simulink model "TosAeb"  (R2021a)
settings: Solver=FixedStepDiscrete  StartTime=0.0  StopTime=inf  FixedStep=0.01  RelTol=1e-3
undefined symbols (not defined in the file or in its folder): ACCModSts, AccSts1, AEB_ObjectMovingOrientation, AEB_ObjectMovingState, CN_BTCActive, CN_CurrentGearR, CN_CurrentGearR_DHT, CN_PTCActive, CN_VDCActive, CN_VSC_EspOff, CN_VSC_FCW_AEB_Error, CN_VSC_FCW_AEB_NoDispay, CN_VSC_FCW_AEB_PerfmDgdtn, CN_VSC_FCW_AEB_TempUnAvlbl, CN_VSC_VehStandstill, CNaccelAvoidMax, CNaccelAvoidMin, CNbrakePedalPress, CNcomfortStrLeftAccelMax, CNcomfortStrLeftAccelMin, CNcomfortStrRightAccelMax, CNcomfortStrRightAccelMin, CNDrvDoorOpen, CNDrvModeEco, CNDrvModeNorm, CNDrvModeOther, CNDrvModeSport, CNDrvSBRreminder, CNNull, CNobjInvalidID, CNPassDoorOpen, CNPassSBRreminder, CNPEBSAccelNegInf, CNPEBSAccelNull, CNPEBSTimeInf, CNPEBSTimeNull, CNSTOPMAX, CNSTOPNULL, CNsubjWidth, CNSwtOFF, CNSwtON, CNTTCMAX, CNTTCNULL, CNVehConfigAEBCycNone, CNVehConfigAEBNone, CNVehConfigAEBPedNone, CNVehConfigFCWNone, CNVehConfigJANone, CollisionSeverity, FALSE, FCWSnvty, JACycObjCalcInfoDefaultParams, JAPedObjCalcInfoDefaultParams, JAVehObjCalcInfoDefaultParams, latCycObjCalcInfoDefaultParams, latPedObjCalcInfoDefaultParams, longObjCalcInfoDefaultParams, ModSts, movingStatus, ObjectType ... (+1112)
libraries: simulink x3031, AEBSFunctionLib x50, Ta_Library x33, AEBSStateMachineLib x23, Mylibrary x12, simulink_extras x7, ObjSelect_lib x3, dspstat3 x2
(part 54 of 102)

== system TosAeb/FcwAebJa_Step_sys/ALG_AEBSystem/ALG_AEBController/AEB_FuncCondCalcApp/PedFunctionCondCalc/pedABASuppAborCond/pedABASuppCondCalc/pedABASituationRelActiveCondCalc/pedABASituationRelSuppCondCalc ==
  latCollisionSeverity : Inport
  subjCurvTraj : Inport  Port=2
  subjLatAccel : Inport  Port=3
  colliisonRiskProb : Inport  Port=4
  ObjectTypeCameraConfirmed : Inport  Port=5
  pedLatVyCond4ABA : Inport  Port=6
  objLongDistCond4ABA : Inport  Port=7
  Compare To Constant1 : simulink/Logic and Bit Operations/Compare To Constant  relop===  const=CollisionSeverity.None  OutDataTypeStr=boolean
  Compare To Constant10 : simulink/Logic and Bit Operations/Compare To Constant  relop=>=  const=P_AEB_Func_pedABAColliisonRiskProbSupThres4Radar  OutDataTypeStr=boolean
  Compare To Constant11 : simulink/Logic and Bit Operations/Compare To Constant  relop===  const=FALSE  OutDataTypeStr=boolean
  Compare To Constant2 : simulink/Logic and Bit Operations/Compare To Constant  relop=>  const=P_AEB_Func_pedABASubjCurvTrajSupThres  OutDataTypeStr=boolean
  Compare To Constant3 : simulink/Logic and Bit Operations/Compare To Constant  relop=>  const=P_AEB_Func_pedABASubjLatAccelSupThres  OutDataTypeStr=boolean
  Compare To Constant4 : simulink/Logic and Bit Operations/Compare To Constant  relop===  const=FALSE  OutDataTypeStr=boolean
  Compare To Constant7 : simulink/Logic and Bit Operations/Compare To Constant  relop===  const=FALSE  OutDataTypeStr=boolean
  Compare To Constant8 : simulink/Logic and Bit Operations/Compare To Constant  relop===  const=TRUE  OutDataTypeStr=boolean
  Compare To Constant9 : simulink/Logic and Bit Operations/Compare To Constant  relop=>=  const=P_AEB_Func_pedABAColliisonRiskProbSupThres4Fusion  OutDataTypeStr=boolean
  Logical Operator1 : Logic  Operator=OR  AllPortsSameDT=off  OutDataTypeStr=boolean
  Logical Operator2 : Logic  Operator=OR  Inputs=5  AllPortsSameDT=off  OutDataTypeStr=boolean
  Switch : Switch  Criteria=u2 ~= 0
  pedABASituationRelSuppCondFulfilled : Outport
  wires:
  Compare To Constant8/1 -> Switch/2
  colliisonRiskProb/1 -> Compare To Constant9/1, Compare To Constant10/1
  Switch/1 -> Compare To Constant11/1
  Compare To Constant10/1 -> Switch/3
  Compare To Constant9/1 -> Switch/1
  ObjectTypeCameraConfirmed/1 -> Compare To Constant8/1
  latCollisionSeverity/1 -> Compare To Constant1/1
  objLongDistCond4ABA/1 -> Compare To Constant7/1
  Compare To Constant4/1 -> Logical Operator2/4  "AEB_PedLatVySuppCond4ABA"
  subjLatAccel/1 -> Compare To Constant3/1
  Compare To Constant7/1 -> Logical Operator2/5  "AEB_PedLongDistSuppCond4ABA"
  Compare To Constant11/1 -> Logical Operator2/3  "pedCritTriggerReleventSuppCondFulfilled"
  Logical Operator1/1 -> Logical Operator2/2  "brakeReleventSuppCondFulfilled"
  Compare To Constant1/1 -> Logical Operator2/1  "pedCollisionSeverityRelSuppCondFulfilled"
  subjCurvTraj/1 -> Compare To Constant2/1
  Logical Operator2/1 -> pedABASituationRelSuppCondFulfilled/1
  Compare To Constant2/1 -> Logical Operator1/1
  pedLatVyCond4ABA/1 -> Compare To Constant4/1
  Compare To Constant3/1 -> Logical Operator1/2

== system TosAeb/FcwAebJa_Step_sys/ALG_AEBSystem/ALG_AEBController/AEB_FuncCondCalcApp/PedFunctionCondCalc/pedABASuppAborCond/pedABASuppCondCalc/pedABASituationRelActiveCondCalc/vyCorrCalc ==
  ObjectTypeCameraConfirmed : Inport
  vy : Inport  Port=2
  Abs : Abs
  Compare To Constant10 : simulink/Logic and Bit Operations/Compare To Constant  relop=>=  const=P_AEB_Func_pedABAVyThres4Radar  OutDataTypeStr=boolean
  Compare To Constant8 : simulink/Logic and Bit Operations/Compare To Constant  relop===  const=TRUE  OutDataTypeStr=boolean
  Compare To Constant9 : simulink/Logic and Bit Operations/Compare To Constant  relop=>=  const=P_AEB_Func_pedABAVyThres4Fusion  OutDataTypeStr=boolean
  Switch : Switch  Criteria=u2 ~= 0
  objLatVyCond4ABA : Outport
  wires:
  Abs/1 -> Compare To Constant10/1, Compare To Constant9/1
  Compare To Constant10/1 -> Switch/3
  ObjectTypeCameraConfirmed/1 -> Compare To Constant8/1
  Compare To Constant8/1 -> Switch/2
  Switch/1 -> objLatVyCond4ABA/1
  Compare To Constant9/1 -> Switch/1
  vy/1 -> Abs/1  "vy"

== system TosAeb/FcwAebJa_Step_sys/ALG_AEBSystem/ALG_AEBController/AEB_FuncCondCalcApp/PedFunctionCondCalc/pedAEBfullSuppAborCond/pedAEBfullSuppCondCalc/pedAEBfullSituationRelSuppCondCalc/dy_ConfirmCalc ==
  note: P_AEB_Func_pedAEBfullDy
  note: [11.1;16.67]
      | [0.12;0.1]
  subjVel : Inport
  dxFrontBumper : Inport  Port=2
  dy : Inport  Port=3
  1-D Lookup Table : Lookup_n-D  NumberOfTableDimensions=1  BreakpointsForDimension1=P_AEB_Func_pedAEBfullDyConfirmX  Table=P_AEB_Func_pedAEBfullDyConfirmY  ExtrapMethod=Clip  InternalRulePriority=Speed  RndMeth=Simplest  InputSameDT=on
  Add : Sum  Inputs=+++
  Constant : Constant  Value=P_AEB_Func_pedAEBfullDyOffset
  Constant1 : Constant  Value=CNsubjWidth
  Gain : Gain  Gain=0.5
  Product : Product
  Relational Operator : RelationalOperator  Operator=<=  OutDataTypeStr=boolean  RndMeth=Simplest
  dy_Confirm : Outport
  wires:
  Relational Operator/1 -> dy_Confirm/1
  Add/1 -> Relational Operator/2
  dy/1 -> Relational Operator/1
  Product/1 -> Add/3
  Constant/1 -> Add/2
  Gain/1 -> Add/1
  Constant1/1 -> Gain/1
  dxFrontBumper/1 -> Product/2
  1-D Lookup Table/1 -> Product/1
  subjVel/1 -> 1-D Lookup Table/1

== system TosAeb/FcwAebJa_Step_sys/ALG_AEBSystem/ALG_AEBController/AEB_FuncCondCalcApp/PedFunctionCondCalc/pedAEBfullSuppAborCond/pedAEBfullSuppCondCalc/pedAEBfullSituationRelSuppCondCalc/latDistCondCalc ==
  dy : Inport
  FcwHMISetting : Inport  Port=2
  subjVel : Inport  Port=3
  vy : Inport  Port=4
  Abs : Abs
  LateralDistanceThresHMISettingEarly : Subsystem  TreatAsAtomicUnit=on
  LateralDistanceThresHMISettingLate : Subsystem  TreatAsAtomicUnit=on
  Merge : Merge
  Relational Operator : RelationalOperator  Operator=<=  OutDataTypeStr=boolean  RndMeth=Simplest
  Switch Case : SwitchCase  CaseConditions={[FCWSnvty.HMISettingEarly,FCWSnvty.HMISettingMiddle]}
  latDistCond : Outport
  wires:
  LateralDistanceThresHMISettingEarly/1(latDistThres) -> Merge/1
  FcwHMISetting/1 -> Switch Case/1
  Abs/1 -> Relational Operator/1
  Switch Case/1 -> LateralDistanceThresHMISettingEarly/Action
  Merge/1 -> Relational Operator/2  "latDistThres"
  dy/1 -> Abs/1
  Relational Operator/1 -> latDistCond/1
  Switch Case/2 -> [HMISettingLate]
  LateralDistanceThresHMISettingLate/1(latDistThres) -> Merge/2
  subjVel/1 -> LateralDistanceThresHMISettingEarly/1(subjVel)
  vy/1 -> LateralDistanceThresHMISettingLate/1(vy)
  [HMISettingLate] -> LateralDistanceThresHMISettingLate/Action

== system TosAeb/FcwAebJa_Step_sys/ALG_AEBSystem/ALG_AEBController/AEB_FuncCondCalcApp/PedFunctionCondCalc/pedAEBfullSuppAborCond/pedAEBfullSuppCondCalc/pedAEBfullSituationRelSuppCondCalc/longDistCond4fullBrkCalc ==
  dxFrontBumper : Inport
  distSecure : Inport  Port=2
  distBraking : Inport  Port=3
  subjVel : Inport  Port=4
  Add : Sum
  Constant : Constant  Value=P_AEB_Func_pedAEBfullLongDistTimeOffset
  Constant1 : Constant  Value=0.1  OutDataTypeStr=single
  Product : Product
  Relational Operator : RelationalOperator  Operator=<=  OutDataTypeStr=boolean  RndMeth=Simplest
  Relational Operator1 : RelationalOperator  Operator=<=  OutDataTypeStr=boolean  RndMeth=Simplest
  Terminator : Terminator
  longDistCond4fullBrk : Outport
  longDistMinCond4Brake : Outport  Port=2
  wires:
  Constant/1 -> Product/2
  subjVel/1 -> Product/1
  dxFrontBumper/1 -> Relational Operator/1, Relational Operator1/1
  Relational Operator1/1 -> longDistMinCond4Brake/1
  Add/1 -> Relational Operator/2
  distBraking/1 -> Add/2
  distSecure/1 -> Add/1
  Relational Operator/1 -> longDistCond4fullBrk/1
  Constant1/1 -> Relational Operator1/2
  Product/1 -> Terminator/1

== system TosAeb/FcwAebJa_Step_sys/ALG_AEBSystem/ALG_AEBController/AEB_FuncCondCalcApp/PedFunctionCondCalc/pedAEBfullSuppAborCond/pedAEBfullSuppCondCalc/pedAEBfullSituationRelSuppCondCalc/objLatVyCond ==
  ObjectTypeCameraConfirmed : Inport
  vy : Inport  Port=2
  dy : Inport  Port=3
  Constant : Constant  Value=P_AEB_Func_pedAEBfullVyMaxThres
  Constant1 : Constant  Value=P_AEB_Func_pedAEBfullDyInnerAreaThres
  Constant2 : Constant  Value=P_AEB_Func_pedAEBfullDyMiddleAreaThres
  Constant3 : Constant  Value=P_AEB_Func_pedAEBfullVyInnerAreaThres4Fusion
  Constant4 : Constant  Value=P_AEB_Func_pedAEBfullVyMiddleAreaThres4Fusion
  Constant5 : Constant  Value=P_AEB_Func_pedAEBfullVyOuterAreaThres4Fusion
  Constant6 : Constant  Value=P_AEB_Func_pedAEBfullVyInnerAreaThres4Radar
  Constant7 : Constant  Value=P_AEB_Func_pedAEBfullVyMiddleAreaThres4Radar
  Constant8 : Constant  Value=P_AEB_Func_pedAEBfullVyOuterAreaThres4Radar
  objLatVyCond4FullBrakeCalc : Stateflow chart  TreatAsAtomicUnit=on
  objLatVyCond4FullBrake : Outport
  wires:
  Constant8/1 -> objLatVyCond4FullBrakeCalc/12(P_pedAEBfullVyOuterAreaThres4Radar)
  Constant7/1 -> objLatVyCond4FullBrakeCalc/11(P_pedAEBfullVyMiddleAreaThres4Radar)
  Constant6/1 -> objLatVyCond4FullBrakeCalc/10(P_pedAEBfullVyInnerAreaThres4Radar)
  Constant5/1 -> objLatVyCond4FullBrakeCalc/9(P_pedAEBfullVyOuterAreaThres4Fusion)
  Constant4/1 -> objLatVyCond4FullBrakeCalc/8(P_pedAEBfullVyMiddleAreaThres4Fusion)
  Constant3/1 -> objLatVyCond4FullBrakeCalc/7(P_pedAEBfullVyInnerAreaThres4Fusion)
  Constant2/1 -> objLatVyCond4FullBrakeCalc/6(P_pedAEBfullDyMiddleAreaThres)
  Constant1/1 -> objLatVyCond4FullBrakeCalc/5(P_pedAEBfullDyInnerAreaThres)
  Constant/1 -> objLatVyCond4FullBrakeCalc/4(P_pedAEBfullVyMaxThres)
  vy/1 -> objLatVyCond4FullBrakeCalc/3(vy)
  ObjectTypeCameraConfirmed/1 -> objLatVyCond4FullBrakeCalc/1(objFusionType)
  dy/1 -> objLatVyCond4FullBrakeCalc/2(dy)
  objLatVyCond4FullBrakeCalc/1(objLatVyCond4FullBrake) -> objLatVyCond4FullBrake/1

== system TosAeb/FcwAebJa_Step_sys/ALG_AEBSystem/ALG_AEBController/AEB_FuncCondCalcApp/PedFunctionCondCalc/pedAEBfullSuppAborCond/pedAEBfullSuppCondCalc/pedAEBfullSituationRelSuppCondCalc/pedAEBfullSituationRelSuppCond ==
  latCollisionSeverity : Inport
  subjCurvTraj : Inport  Port=2
  subjLatAccel : Inport  Port=3
  pedCollisionProbFlg : Inport  Port=4
  pedCollisionRisk : Inport  Port=5
  ObjectTypeCameraConfirmed : Inport  Port=6
  objLatVyCond4FullBrake : Inport  Port=7
  latDistCond : Inport  Port=8
  objLongDistCond4FullBrake : Inport  Port=9
  distMinCond4FullBrake : Inport  Port=10
  Compare To Constant : simulink/Logic and Bit Operations/Compare To Constant  relop===  const=TRUE  OutDataTypeStr=boolean
  Compare To Constant1 : simulink/Logic and Bit Operations/Compare To Constant  relop===  const=CollisionSeverity.None  OutDataTypeStr=boolean
  Compare To Constant{4,6..7,9..10} (5 alike) : simulink/Logic and Bit Operations/Compare To Constant  relop===  const=FALSE  OutDataTypeStr=boolean
  Compare To Constant11 : simulink/Logic and Bit Operations/Compare To Constant  relop===  const=TRUE  OutDataTypeStr=boolean
  Compare To Constant12 : simulink/Logic and Bit Operations/Compare To Constant  relop=>  const=P_AEB_Func_pedAEBfullColliisonRiskProbSupThres4Fusion  OutDataTypeStr=boolean
  Compare To Constant13 : simulink/Logic and Bit Operations/Compare To Constant  relop=>  const=P_AEB_Func_pedAEBfullColliisonRiskProbSupThres4Radar  OutDataTypeStr=boolean
  Compare To Constant2 : simulink/Logic and Bit Operations/Compare To Constant  relop=>  const=P_AEB_Func_pedAEBfullSubjCurvTrajSupThres  OutDataTypeStr=boolean
  Compare To Constant3 : simulink/Logic and Bit Operations/Compare To Constant  relop=>  const=P_AEB_Func_pedAEBfullSubjLatAccelSupThres  OutDataTypeStr=boolean
  Compare To Constant5 : simulink/Logic and Bit Operations/Compare To Constant  relop===  const=CollisionSeverity.Low  OutDataTypeStr=boolean
  Compare To Constant8 : simulink/Logic and Bit Operations/Compare To Constant  relop===  const=CollisionSeverity.Medium  OutDataTypeStr=boolean
  Logical Operator1 : Logic  Operator=OR  AllPortsSameDT=off  OutDataTypeStr=boolean
  Logical Operator2 : Logic  Operator=OR  Inputs=7  AllPortsSameDT=off  OutDataTypeStr=boolean
  Logical Operator3 : Logic  Operator=OR  Inputs=3  AllPortsSameDT=off  OutDataTypeStr=boolean
  Logical Operator4 : Logic  Operator=OR  AllPortsSameDT=off  OutDataTypeStr=boolean
  Switch : Switch  Criteria=u2 ~= 0
  pedAEBfullSituationRelSuppCondFulfilled : Outport
  wires:
  Compare To Constant13/1 -> Switch/3
  Compare To Constant12/1 -> Switch/1
  Switch/1 -> Compare To Constant6/1
  pedCollisionRisk/1 -> Compare To Constant12/1, Compare To Constant13/1
  Compare To Constant/1 -> Switch/2
  ObjectTypeCameraConfirmed/1 -> Compare To Constant/1
  Compare To Constant11/1 -> Logical Operator2/7  "AEB_PedLongDistMinSuppCond4FullBrake"
  Compare To Constant7/1 -> Logical Operator2/6  "AEB_PedLongDistSuppCond4FullBrake"
  Compare To Constant9/1 -> Logical Operator2/5  "AEB_PedLatDistSuppCond4FullBrake"
  distMinCond4FullBrake/1 -> Compare To Constant11/1
  latCollisionSeverity/1 -> Compare To Constant8/1, Compare To Constant5/1, Compare To Constant1/1
  objLongDistCond4FullBrake/1 -> Compare To Constant7/1
  Compare To Constant4/1 -> Logical Operator2/4  "AEB_PedLatVySuppCond4FullBrake"
  subjLatAccel/1 -> Compare To Constant3/1
  Logical Operator4/1 -> Logical Operator2/3  "pedCritTriggerReleventSuppCondFulfilled"
  Logical Operator1/1 -> Logical Operator2/2  "SubjMovingReleventSuppCondFulfilled"
  Logical Operator3/1 -> Logical Operator2/1  "pedCollisionDgrLvlRelSuppCondFulfilled"
  subjCurvTraj/1 -> Compare To Constant2/1
  Logical Operator2/1 -> pedAEBfullSituationRelSuppCondFulfilled/1
  Compare To Constant2/1 -> Logical Operator1/1
  objLatVyCond4FullBrake/1 -> Compare To Constant4/1
  Compare To Constant3/1 -> Logical Operator1/2
  Compare To Constant1/1 -> Logical Operator3/1
  Compare To Constant5/1 -> Logical Operator3/2
  Compare To Constant8/1 -> Logical Operator3/3
  latDistCond/1 -> Compare To Constant9/1
  pedCollisionProbFlg/1 -> Compare To Constant10/1
  Compare To Constant6/1 -> Logical Operator4/2
  Compare To Constant10/1 -> Logical Operator4/1

== system TosAeb/FcwAebJa_Step_sys/ALG_AEBSystem/ALG_AEBController/AEB_FuncCondCalcApp/PedFunctionCondCalc/pedAEBfullSuppAborCond/pedAEBfullSuppCondCalc/pedAEBfullSituationRelSuppCondCalc/vy_ConfirmCalc ==
  vy : Inport
  dy : Inport  Port=2
  1-D Lookup Table : Lookup_n-D  NumberOfTableDimensions=1  BreakpointsForDimension1=[0.45;2]  Table=[0.6;1.15]  ExtrapMethod=Clip  InternalRulePriority=Speed  RndMeth=Simplest  InputSameDT=on
  Relational Operator : RelationalOperator  Operator=>  OutDataTypeStr=boolean  RndMeth=Simplest
  vy_Confirm : Outport
  wires:
  dy/1 -> 1-D Lookup Table/1
  vy/1 -> Relational Operator/1
  Relational Operator/1 -> vy_Confirm/1
  1-D Lookup Table/1 -> Relational Operator/2

== system TosAeb/FcwAebJa_Step_sys/ALG_AEBSystem/ALG_AEBController/AEB_FuncCondCalcApp/PedFunctionCondCalc/pedAEBfullSuppAborCond/pedAEBfullSuppCondCalc/pedAEBfullVehRelSuppCondCalc/brakeReleventSuppCond ==
  VehConfigIbooster : Inport
  ESP_MasterCylindBrakePressValid : Inport  Port=2
  ESP_MasterCylindBrakePress : Inport  Port=3
  Compare To Constant : simulink/Logic and Bit Operations/Compare To Constant  relop===  const=FALSE  OutDataTypeStr=boolean
  If : If  IfExpression=u1
  Merge : Merge
  brakeReleventSuppCond_ESP : Subsystem  VariantControl=(default)
  brakeReleventSuppCond_IBooster : Subsystem  VariantControl=P_AEB_PowerTrainConfig == 1
  brakeReleventSuppCond : Outport
  wires:
  ESP_MasterCylindBrakePress/1 -> brakeReleventSuppCond_ESP/2(ESP_MasterCylindBrakePress)
  ESP_MasterCylindBrakePressValid/1 -> brakeReleventSuppCond_ESP/1(ESP_MasterCylindBrakePressValid)
  Merge/1 -> brakeReleventSuppCond/1
  brakeReleventSuppCond_IBooster/1(brakeReleventSuppCond) -> Merge/2
  brakeReleventSuppCond_ESP/1(brakeReleventSuppCond) -> Merge/1
  If/2 -> brakeReleventSuppCond_IBooster/Action
  If/1 -> brakeReleventSuppCond_ESP/Action
  Compare To Constant/1 -> If/1
  VehConfigIbooster/1 -> Compare To Constant/1

== system TosAeb/FcwAebJa_Step_sys/ALG_AEBSystem/ALG_AEBController/AEB_FuncCondCalcApp/PedFunctionCondCalc/pedPreWarningSuppAborCond/pedPreWarningSuppCondCalc/pedPreWarningSituationRelActiveCondCalc/PreWarningMaxTimeCalc ==
  FcwHMISetting : Inport
  subjVel : Inport  Port=2
  dxFrontBumper : Inport  Port=3
  Compare To Constant : simulink/Logic and Bit Operations/Compare To Constant  relop===  const=FCWSnvty.HMISettingEarly  OutDataTypeStr=boolean
  Compare To Constant1 : simulink/Logic and Bit Operations/Compare To Constant  relop===  const=FCWSnvty.HMISettingMiddle  OutDataTypeStr=boolean
  Constant : Constant  Value=P_AEB_Func_pedPrewarningLongDistTimeOffsetHmiEarlyMid
  Constant1 : Constant  Value=P_AEB_Func_pedPrewarningLongDistTimeOffsetHmiLate
  Logical Operator : Logic  Operator=OR  AllPortsSameDT=off  OutDataTypeStr=boolean
  Product : Product
  Relational Operator : RelationalOperator  Operator=<=  OutDataTypeStr=boolean  RndMeth=Simplest
  Switch : Switch  Criteria=u2 ~= 0
  longDistCond4PreWarning : Outport
  wires:
  FcwHMISetting/1 -> Compare To Constant1/1, Compare To Constant/1
  Compare To Constant/1 -> Logical Operator/1
  Compare To Constant1/1 -> Logical Operator/2
  Logical Operator/1 -> Switch/2
  Constant/1 -> Switch/1
  Constant1/1 -> Switch/3
  Switch/1 -> Product/1
  subjVel/1 -> Product/2
  Product/1 -> Relational Operator/2
  dxFrontBumper/1 -> Relational Operator/1
  Relational Operator/1 -> longDistCond4PreWarning/1

== system TosAeb/FcwAebJa_Step_sys/ALG_AEBSystem/ALG_AEBController/AEB_FuncCondCalcApp/PedFunctionCondCalc/pedPreWarningSuppAborCond/pedPreWarningSuppCondCalc/pedPreWarningSituationRelActiveCondCalc/longDistMinCond4PreWarningCalc ==
  dxFrontBumper : Inport
  subjVel : Inport  Port=2
  Constant : Constant
  Product : Product
  Relational Operator : RelationalOperator  Operator=<=  OutDataTypeStr=boolean  RndMeth=Simplest
  longDistMinCond4PreWarning : Outport
  wires:
  Relational Operator/1 -> longDistMinCond4PreWarning/1
  dxFrontBumper/1 -> Relational Operator/1
  subjVel/1 -> Product/1
  Constant/1 -> Product/2
  Product/1 -> Relational Operator/2

== system TosAeb/FcwAebJa_Step_sys/ALG_AEBSystem/ALG_AEBController/AEB_FuncCondCalcApp/PedFunctionCondCalc/pedPreWarningSuppAborCond/pedPreWarningSuppCondCalc/pedPreWarningSituationRelActiveCondCalc/pedPreWarningSituationRelSuppCond ==
  latCollisionSeverity : Inport
  subjCurvTraj : Inport  Port=2
  subjLatAccel : Inport  Port=3
  colliisonRiskProb : Inport  Port=4
  ObjectTypeCameraConfirmed : Inport  Port=5
  pedLatVyCond4Prewarning : Inport  Port=6
  longDistCond4PreWarning : Inport  Port=7
  longDistMinCond4PreWarning : Inport  Port=8
  Compare To Constant1 : simulink/Logic and Bit Operations/Compare To Constant  relop===  const=CollisionSeverity.None  OutDataTypeStr=boolean
  Compare To Constant10 : simulink/Logic and Bit Operations/Compare To Constant  relop=>=  const=P_AEB_Func_pedPrewarningColliisonRiskProbSupThres4Radar  OutDataTypeStr=boolean
  Compare To Constant{4..5,7,11} (4 alike) : simulink/Logic and Bit Operations/Compare To Constant  relop===  const=FALSE  OutDataTypeStr=boolean
  Compare To Constant2 : simulink/Logic and Bit Operations/Compare To Constant  relop=>  const=P_AEB_Func_pedPrewarningSubjCurvTrajSupThres  OutDataTypeStr=boolean
  Compare To Constant3 : simulink/Logic and Bit Operations/Compare To Constant  relop=>  const=P_AEB_Func_pedPrewarningSubjLatAccelSupThres  OutDataTypeStr=boolean
  Compare To Constant8 : simulink/Logic and Bit Operations/Compare To Constant  relop===  const=TRUE  OutDataTypeStr=boolean
  Compare To Constant9 : simulink/Logic and Bit Operations/Compare To Constant  relop=>=  const=P_AEB_Func_pedPrewarningColliisonRiskProbSupThres4Fusion  OutDataTypeStr=boolean
  Logical Operator1 : Logic  Operator=OR  AllPortsSameDT=off  OutDataTypeStr=boolean
  Logical Operator2 : Logic  Operator=OR  Inputs=6  AllPortsSameDT=off  OutDataTypeStr=boolean
  Switch : Switch  Criteria=u2 ~= 0
  pedPreWarningSituationRelSuppCondFulfilled : Outport
  wires:
  Compare To Constant5/1 -> Logical Operator2/6  "AEB_PedLongDistMinSuppCond4PreWarning"
  Compare To Constant7/1 -> Logical Operator2/5  "AEB_PedLongDistSuppCond4PreWarning"
  Compare To Constant4/1 -> Logical Operator2/4  "AEB_PedLatVySuppCond4Prewarning"
  Compare To Constant11/1 -> Logical Operator2/3
  Compare To Constant10/1 -> Switch/3
  Switch/1 -> Compare To Constant11/1
  ObjectTypeCameraConfirmed/1 -> Compare To Constant8/1
  longDistCond4PreWarning/1 -> Compare To Constant7/1
  longDistMinCond4PreWarning/1 -> Compare To Constant5/1
  Compare To Constant9/1 -> Switch/1
  Compare To Constant8/1 -> Switch/2
  colliisonRiskProb/1 -> Compare To Constant10/1, Compare To Constant9/1
  Compare To Constant3/1 -> Logical Operator1/2
  pedLatVyCond4Prewarning/1 -> Compare To Constant4/1
  Compare To Constant2/1 -> Logical Operator1/1
  Logical Operator2/1 -> pedPreWarningSituationRelSuppCondFulfilled/1
  subjCurvTraj/1 -> Compare To Constant2/1
  Compare To Constant1/1 -> Logical Operator2/1
  Logical Operator1/1 -> Logical Operator2/2
  subjLatAccel/1 -> Compare To Constant3/1
  latCollisionSeverity/1 -> Compare To Constant1/1

== system TosAeb/FcwAebJa_Step_sys/ALG_AEBSystem/ALG_AEBController/AEB_FuncCondCalcApp/PedFunctionCondCalc/pedPreWarningSuppAborCond/pedPreWarningSuppCondCalc/pedPreWarningSituationRelActiveCondCalc/vyCorrCalc ==
  ObjectTypeCameraConfirmed : Inport
  vy : Inport  Port=2
  Abs : Abs
  Compare To Constant10 : simulink/Logic and Bit Operations/Compare To Constant  relop=>=  const=P_AEB_Func_pedPrewarningVyhres4Radar  OutDataTypeStr=boolean
  Compare To Constant8 : simulink/Logic and Bit Operations/Compare To Constant  relop===  const=TRUE  OutDataTypeStr=boolean
  Compare To Constant9 : simulink/Logic and Bit Operations/Compare To Constant  relop=>=  const=P_AEB_Func_pedPrewarningVyThres4Fusion  OutDataTypeStr=boolean
  Switch : Switch  Criteria=u2 ~= 0
  objLatVyCond4Prewarning : Outport
  wires:
  Abs/1 -> Compare To Constant10/1, Compare To Constant9/1
  Compare To Constant10/1 -> Switch/3
  ObjectTypeCameraConfirmed/1 -> Compare To Constant8/1
  Compare To Constant8/1 -> Switch/2
  Switch/1 -> objLatVyCond4Prewarning/1
  Compare To Constant9/1 -> Switch/1
  vy/1 -> Abs/1  "vy"

== system TosAeb/FcwAebJa_Step_sys/ALG_AEBSystem/ALG_AEBController/AEB_FuncCondCalcApp/PedFunctionCondCalc/pedPreWarningSuppAborCond/pedPreWarningSuppCondCalc/pedPreWarningVehRelActiveCondCalc/brakeReleventSuppCond ==
  VehConfigIbooster : Inport
  ESP_MasterCylindBrakePressValid : Inport  Port=2
  ESP_MasterCylindBrakePress : Inport  Port=3
  IB_BrkPedalAppld : Inport  Port=4
  Compare To Constant : simulink/Logic and Bit Operations/Compare To Constant  relop===  const=FALSE  OutDataTypeStr=boolean
  If : If  IfExpression=u1
  Merge : Merge
  brakeReleventSuppCond_ESP : Subsystem  VariantControl=(default)
  brakeReleventSuppCond_IBooster : Subsystem  VariantControl=P_AEB_PowerTrainConfig == 1
  brakeReleventSuppCond : Outport
  wires:
  ESP_MasterCylindBrakePress/1 -> brakeReleventSuppCond_ESP/2(ESP_MasterCylindBrakePress)
  ESP_MasterCylindBrakePressValid/1 -> brakeReleventSuppCond_ESP/1(ESP_MasterCylindBrakePressValid)
  IB_BrkPedalAppld/1 -> brakeReleventSuppCond_IBooster/1(IB_BrkPedalAppld)
  Merge/1 -> brakeReleventSuppCond/1
  brakeReleventSuppCond_IBooster/1(brakeReleventSuppCond) -> Merge/2
  brakeReleventSuppCond_ESP/1(brakeReleventSuppCond) -> Merge/1
  If/2 -> brakeReleventSuppCond_IBooster/Action
  If/1 -> brakeReleventSuppCond_ESP/Action
  Compare To Constant/1 -> If/1
  VehConfigIbooster/1 -> Compare To Constant/1

== system TosAeb/FcwAebJa_Step_sys/ALG_AEBSystem/ALG_AEBController/AEB_FuncCondCalcApp/PedFunctionCondCalc/pedPrefillSuppAborCond/pedPrefillSuppCondCalc/pedPrefillSituationRelActiveCondCalc/objLatVyCond4PrefillCalc ==
  objObjectTypeCameraConfirmed : Inport
  dy : Inport  Port=2
  vy : Inport  Port=3
  Constant : Constant  Value=P_AEB_Func_pedPrefillVyMaxThres
  Constant1 : Constant  Value=P_AEB_Func_pedPrefillDyInnerAreaThres
  Constant2 : Constant  Value=P_AEB_Func_pedPrefillDyMiddleAreaThres
  Constant3 : Constant  Value=P_AEB_Func_pedPrefillVyInnerAreaThres4Fusion
  Constant4 : Constant  Value=P_AEB_Func_pedPrefillVyInnerAreaThres4Radar
  Constant5 : Constant  Value=P_AEB_Func_pedPrefillVyMiddleAreaThres4Fusion
  Constant6 : Constant  Value=P_AEB_Func_pedPrefillVyMiddleAreaThres4Radar
  Constant7 : Constant  Value=P_AEB_Func_pedPrefillVyOuterAreaThres4Fusion
  Constant8 : Constant  Value=P_AEB_Func_pedPrefillVyOuterAreaThres4Radar
  ObjectIsLaterallyMovingForPrefillCondCalc : Stateflow chart  TreatAsAtomicUnit=on
  ObjLatVyCond4Prefill : Outport
  wires:
  Constant8/1 -> ObjectIsLaterallyMovingForPrefillCondCalc/12(P_pedPrefillVyOuterAreaThres4Radar)
  Constant7/1 -> ObjectIsLaterallyMovingForPrefillCondCalc/11(P_pedPrefillVyOuterAreaThres4Fusion)
  Constant6/1 -> ObjectIsLaterallyMovingForPrefillCondCalc/10(P_pedPrefillVyMiddleAreaThres4Radar)
  Constant5/1 -> ObjectIsLaterallyMovingForPrefillCondCalc/9(P_pedPrefillVyMiddleAreaThres4Fusion)
  Constant4/1 -> ObjectIsLaterallyMovingForPrefillCondCalc/8(P_pedPrefillVyInnerAreaThres4Radar)
  Constant3/1 -> ObjectIsLaterallyMovingForPrefillCondCalc/7(P_pedPrefillVyInnerAreaThres4Fusion)
  Constant2/1 -> ObjectIsLaterallyMovingForPrefillCondCalc/6(P_pedPrefillDyMiddleAreaThres)
  Constant1/1 -> ObjectIsLaterallyMovingForPrefillCondCalc/5(P_pedPrefillDyInnerAreaThres)
  Constant/1 -> ObjectIsLaterallyMovingForPrefillCondCalc/4(P_pedPrefillVyMaxThres)
  objObjectTypeCameraConfirmed/1 -> ObjectIsLaterallyMovingForPrefillCondCalc/1(ObjectTypeCameraConfirmed)
  vy/1 -> ObjectIsLaterallyMovingForPrefillCondCalc/3(vyCorr)
  dy/1 -> ObjectIsLaterallyMovingForPrefillCondCalc/2(dy)
  ObjectIsLaterallyMovingForPrefillCondCalc/1(objLatVyCond4Prefill) -> ObjLatVyCond4Prefill/1  "objLatVyCond4Prefill"

== system TosAeb/FcwAebJa_Step_sys/ALG_AEBSystem/ALG_AEBController/AEB_FuncCondCalcApp/PedFunctionCondCalc/pedPrefillSuppAborCond/pedPrefillSuppCondCalc/pedPrefillSituationRelActiveCondCalc/objLongDistCond4PrefillCalc ==
  dxFrontBumper : Inport
  longDist4Brk : Inport  Port=2
  distSecure : Inport  Port=3
  subVel : Inport  Port=4
  Add : Sum  Inputs=3
  Constant : Constant  Value=P_AEB_Func_pedPrefillLongDistTimeOffset
  Product : Product
  Relational Operator : RelationalOperator  Operator=<=  OutDataTypeStr=boolean  RndMeth=Simplest
  ObjLongDistCond4Prefill : Outport
  wires:
  Constant/1 -> Product/2
  subVel/1 -> Product/1
  Add/1 -> Relational Operator/2
  distSecure/1 -> Add/2
  longDist4Brk/1 -> Add/1
  Product/1 -> Add/3
  dxFrontBumper/1 -> Relational Operator/1
  Relational Operator/1 -> ObjLongDistCond4Prefill/1

== system TosAeb/FcwAebJa_Step_sys/ALG_AEBSystem/ALG_AEBController/AEB_FuncCondCalcApp/PedFunctionCondCalc/pedPrefillSuppAborCond/pedPrefillSuppCondCalc/pedPrefillSituationRelActiveCondCalc/pedPrefillSituationRelSuppCond ==
  latCollisionSeverity : Inport
  subjCurvTraj : Inport  Port=2
  subjLatAccel : Inport  Port=3
  colliisonRiskProb : Inport  Port=4
  ObjectTypeCameraConfirmed : Inport  Port=5
  objLongDistCond4Prefill : Inport  Port=6
  objLatVyCond4Prefill : Inport  Port=7
  Compare To Constant1 : simulink/Logic and Bit Operations/Compare To Constant  relop===  const=CollisionSeverity.None  OutDataTypeStr=boolean
  Compare To Constant10 : simulink/Logic and Bit Operations/Compare To Constant  relop=>  const=P_AEB_Func_pedPrefillColliisonRiskProbSupThres4Radar  OutDataTypeStr=boolean
  Compare To Constant2 : simulink/Logic and Bit Operations/Compare To Constant  relop=>  const=P_AEB_Func_pedPrefillSubjCurvTrajSupThres  OutDataTypeStr=boolean
  Compare To Constant3 : simulink/Logic and Bit Operations/Compare To Constant  relop=>  const=P_AEB_Func_pedPrefillSubjLatAccelSupThres  OutDataTypeStr=boolean
  Compare To Constant4 : simulink/Logic and Bit Operations/Compare To Constant  relop===  const=FALSE  OutDataTypeStr=boolean
  Compare To Constant5 : simulink/Logic and Bit Operations/Compare To Constant  relop===  const=CollisionSeverity.Low  OutDataTypeStr=boolean
  Compare To Constant6 : simulink/Logic and Bit Operations/Compare To Constant  relop===  const=FALSE  OutDataTypeStr=boolean
  Compare To Constant7 : simulink/Logic and Bit Operations/Compare To Constant  relop===  const=FALSE  OutDataTypeStr=boolean
  Compare To Constant8 : simulink/Logic and Bit Operations/Compare To Constant  relop===  const=TRUE  OutDataTypeStr=boolean
  Compare To Constant9 : simulink/Logic and Bit Operations/Compare To Constant  relop=>  const=P_AEB_Func_pedPrefillColliisonRiskProbSupThres4Fusion  OutDataTypeStr=boolean
  Logical Operator1 : Logic  Operator=OR  AllPortsSameDT=off  OutDataTypeStr=boolean
  Logical Operator2 : Logic  Operator=OR  Inputs=5  AllPortsSameDT=off  OutDataTypeStr=boolean
  Logical Operator3 : Logic  Operator=OR  AllPortsSameDT=off  OutDataTypeStr=boolean
  Switch : Switch  Criteria=u2 ~= 0
  pedPrefillSituationRelActiveCondFulfilled : Outport
  wires:
  colliisonRiskProb/1 -> Compare To Constant9/1, Compare To Constant10/1
  Compare To Constant9/1 -> Switch/1
  Compare To Constant5/1 -> Logical Operator3/2
  Compare To Constant3/1 -> Logical Operator1/2
  Compare To Constant1/1 -> Logical Operator3/1
  objLatVyCond4Prefill/1 -> Compare To Constant4/1
  Compare To Constant2/1 -> Logical Operator1/1
  Logical Operator2/1 -> pedPrefillSituationRelActiveCondFulfilled/1  "situaitonSuppCond"
  subjCurvTraj/1 -> Compare To Constant2/1
  Logical Operator3/1 -> Logical Operator2/1
  Logical Operator1/1 -> Logical Operator2/2
  Compare To Constant6/1 -> Logical Operator2/3
  Compare To Constant7/1 -> Logical Operator2/4  "AEB_PedLatVySuppCond4Prefill"
  subjLatAccel/1 -> Compare To Constant3/1
  Compare To Constant4/1 -> Logical Operator2/5  "AEB_PedLongDistSuppCond4Prefill"
  objLongDistCond4Prefill/1 -> Compare To Constant7/1
  latCollisionSeverity/1 -> Compare To Constant1/1, Compare To Constant5/1
  Switch/1 -> Compare To Constant6/1
  Compare To Constant10/1 -> Switch/3
  Compare To Constant8/1 -> Switch/2
  ObjectTypeCameraConfirmed/1 -> Compare To Constant8/1

== system TosAeb/FcwAebJa_Step_sys/ALG_AEBSystem/ALG_AEBController/AEB_FuncCondCalcApp/PedFunctionCondCalc/pedPrefillSuppAborCond/pedPrefillSuppCondCalc/pedPrefillVehRelActiveCondCalc/brakeReleventSuppCond ==
  VehConfigIbooster : Inport
  ESP_MasterCylindBrakePressValid : Inport  Port=2
  ESP_MasterCylindBrakePress : Inport  Port=3
  IB_BrkPedalAppld : Inport  Port=4
  Compare To Constant : simulink/Logic and Bit Operations/Compare To Constant  relop===  const=FALSE  OutDataTypeStr=boolean
  If : If  IfExpression=u1
  Merge : Merge
  brakeReleventSuppCond_ESP : Subsystem  VariantControl=(default)
  brakeReleventSuppCond_IBooster : Subsystem  VariantControl=P_AEB_PowerTrainConfig == 1
  brakeReleventSuppCond : Outport
  wires:
  ESP_MasterCylindBrakePress/1 -> brakeReleventSuppCond_ESP/2(ESP_MasterCylindBrakePress)
  ESP_MasterCylindBrakePressValid/1 -> brakeReleventSuppCond_ESP/1(ESP_MasterCylindBrakePressValid)
  IB_BrkPedalAppld/1 -> brakeReleventSuppCond_IBooster/1(IB_BrkPedalAppld)
  Merge/1 -> brakeReleventSuppCond/1
  brakeReleventSuppCond_IBooster/1(brakeReleventSuppCond) -> Merge/2
  brakeReleventSuppCond_ESP/1(brakeReleventSuppCond) -> Merge/1
  If/2 -> brakeReleventSuppCond_IBooster/Action
  If/1 -> brakeReleventSuppCond_ESP/Action
  Compare To Constant/1 -> If/1
  VehConfigIbooster/1 -> Compare To Constant/1

== system TosAeb/FcwAebJa_Step_sys/ALG_AEBSystem/ALG_AEBController/AEB_FuncImpManagementApp/FuncManeuArbCalc/BrkReadyManeuArbCalc/BrkReadyTriggerJudge/ABALvlCalc/If Action Subsystem ==
  ABATrigger : Inport
  longCollisionSeverity : Inport  Port=2
  pedCollisionSeverity : Inport  Port=3
  pedABAFuncImpArb : Inport  Port=4
  Action Port : ActionPort  ActionPortLabel=if(u1)
  ABALvlCalcFlow : Stateflow chart  TreatAsAtomicUnit=on
  Compare To Constant5 : simulink/Logic and Bit Operations/Compare To Constant  relop===  const=ModSts.ActiveState  OutDataTypeStr=boolean
  Switch : Switch  Criteria=u2 ~= 0
  ABALvl : Outport
  wires:
  Compare To Constant5/1 -> Switch/2
  pedABAFuncImpArb/1 -> Compare To Constant5/1
  Switch/1 -> ABALvlCalcFlow/2(AEB_CollisionSeverity)
  ABALvlCalcFlow/1(ABALvl) -> ABALvl/1
  ABATrigger/1 -> ABALvlCalcFlow/1(ABATrigger)
  pedCollisionSeverity/1 -> Switch/1
  longCollisionSeverity/1 -> Switch/3

== system TosAeb/FcwAebJa_Step_sys/ALG_AEBSystem/ALG_AEBController/AEB_FuncImpManagementApp/FuncManeuArbCalc/BrkReadyManeuArbCalc/BrkReadyTriggerJudge/ABALvlCalc/If Action Subsystem1 ==
  ABATrigger : Inport
  FCTB_ABA_Level : Inport  Port=2
  Action Port : ActionPort  ActionPortLabel=elseif(u2)
  Compare To Constant : simulink/Logic and Bit Operations/Compare To Constant  relop===  const=TRUE  OutDataTypeStr=boolean
  Constant : Constant  Value=0  OutDataTypeStr=uint8
  Switch : Switch  Criteria=u2 ~= 0
  ABALvl : Outport
  wires:
  Switch/1 -> ABALvl/1
  Constant/1 -> Switch/3
  FCTB_ABA_Level/1 -> Switch/1
  Compare To Constant/1 -> Switch/2
  ABATrigger/1 -> Compare To Constant/1

== system TosAeb/FcwAebJa_Step_sys/ALG_AEBSystem/ALG_AEBController/AEB_FuncImpManagementApp/FuncManeuArbCalc/BrkReadyManeuArbCalc/BrkReadyTriggerJudge/ABALvlCalc/If Action Subsystem2 ==
  Action Port : ActionPort  ActionPortLabel=else
  Constant : Constant  Value=0  OutDataTypeStr=uint8
  ABALvl : Outport
  wires:
  Constant/1 -> ABALvl/1

== system TosAeb/FcwAebJa_Step_sys/ALG_AEBSystem/ALG_AEBController/AEB_FuncImpManagementApp/FuncManeuArbCalc/brkFuncManeuArbCalc/axTgtReqValueCalc1/AebfullTriggerInfoCalc/AEBControllerInfo ==
  vxPredictedAtStart : Inport
  accelMinIn : Inport  Port=2  OutDataTypeStr=single
  JerkFuncDecMaxIn : Inport  Port=3  OutDataTypeStr=single
  Compare To Constant : simulink/Logic and Bit Operations/Compare To Constant  relop=>=  const=P_AEB_FIM_vxThres_accelMin4lowvRel  OutDataTypeStr=boolean
  Constant : Constant  Value=P_AEB_FIM_accelMin4lowvRel_Config
  Constant1 : Constant  Value=P_AEB_FIM_accelMin4lowvRel  OutDataTypeStr=single
  Constant2 : Constant  Value=P_AEB_FIM_accelMax  OutDataTypeStr=single
  Logical Operator : Logic  AllPortsSameDT=off  OutDataTypeStr=boolean
  Max : MinMax  Function=max  Inputs=2
  Switch : Switch  Criteria=u2 ~= 0
  accelMin : Outport
  accelMax : Outport  Port=2
  JerkFuncDecMax : Outport  Port=3
  wires:
  JerkFuncDecMaxIn/1 -> JerkFuncDecMax/1
  accelMinIn/1 -> Switch/3, Max/1
  Constant1/1 -> Max/2
  Switch/1 -> accelMin/1
  Max/1 -> Switch/1
  Logical Operator/1 -> Switch/2
  Constant/1 -> Logical Operator/2
  Compare To Constant/1 -> Logical Operator/1
  vxPredictedAtStart/1 -> Compare To Constant/1
  Constant2/1 -> accelMax/1

== system TosAeb/FcwAebJa_Step_sys/ALG_AEBSystem/ALG_AEBController/AEB_FuncImpManagementApp/FuncManeuArbCalc/brkFuncManeuArbCalc/axTgtReqValueCalc1/AebfullTriggerInfoCalc/AEBTriggerRelCalc ==
  AebActive : Inport
  vxPredicted : Inport  Port=2
  VSC_VehStandstill : Inport  Port=3
  subjVel : Inport  Port=4
  AEBTriggerInfo : Subsystem
  FuncActvationTimeCalc : Subsystem
  FuncActvationTime : Outport
  AebFullFuncStatus : Outport  Port=2
  AebActiveFirstCycle : Outport  Port=3
  FuncActvationTimeAtStart : Outport  Port=4
  subjVelAtStart : Outport  Port=5
  dxSecureAtStart : Outport  Port=6
  vxPredictedAtStart : Outport  Port=7
  wires:
  AebActive/1 -> FuncActvationTimeCalc/1(AebActive), AEBTriggerInfo/2(AebActive)
  vxPredicted/1 -> AEBTriggerInfo/4(vxPredicted)
  subjVel/1 -> AEBTriggerInfo/3(subjVel)
  AEBTriggerInfo/4(dxSecureAtStart) -> dxSecureAtStart/1
  AEBTriggerInfo/5(subjVelAtStart) -> subjVelAtStart/1
  AEBTriggerInfo/6(vxPredictedAtStart) -> vxPredictedAtStart/1
  AEBTriggerInfo/3(FuncActvationTimeAtStart) -> FuncActvationTimeAtStart/1
  FuncActvationTimeCalc/1(FuncActvationTime) -> AEBTriggerInfo/1(FuncActvationTime), FuncActvationTime/1  "FuncActvationTime"
  VSC_VehStandstill/1 -> AEBTriggerInfo/5(VSC_VehStandstill)
  AEBTriggerInfo/2(AebActiveFirstCycle) -> AebActiveFirstCycle/1
  AEBTriggerInfo/1(AebFullFuncStatus) -> AebFullFuncStatus/1

== system TosAeb/FcwAebJa_Step_sys/ALG_AEBSystem/ALG_AEBController/AEB_FuncImpManagementApp/FuncManeuArbCalc/brkFuncManeuArbCalc/axTgtReqValueCalc1/AebfullTriggerInfoCalc/AebActive ==
  AEBfullFuncImpArb : Inport  OutDataTypeStr=Enum: ModSts
  Compare To Constant : simulink/Logic and Bit Operations/Compare To Constant  relop===  const=ModSts.ActiveState  OutDataTypeStr=boolean
  AebActive : Outport
  wires:
  AEBfullFuncImpArb/1 -> Compare To Constant/1
  Compare To Constant/1 -> AebActive/1

== system TosAeb/FcwAebJa_Step_sys/ALG_AEBSystem/ALG_AEBController/AEB_FuncImpManagementApp/FuncManeuArbCalc/brkFuncManeuArbCalc/axTgtReqValueCalc1/AebfullTriggerInfoCalc/BrakeInfo ==
  subjVel : Inport
  ObjectMovingState : Inport  Port=2
  Compare To Constant : simulink/Logic and Bit Operations/Compare To Constant  relop===  const=AEB_ObjectMovingState.Still  OutDataTypeStr=boolean
  If : If  IfExpression=u1
  If Action Subsystem : Subsystem  TreatAsAtomicUnit=on
  If Action Subsystem1 : Subsystem  TreatAsAtomicUnit=on
  Merge : Merge
  Merge1 : Merge
  accelMin : Outport
  JerkFuncDecMax : Outport  Port=2
  wires:
  Merge/1 -> JerkFuncDecMax/1
  Merge1/1 -> accelMin/1
  If Action Subsystem1/2(accelMin) -> Merge1/2
  If Action Subsystem/2(accelMin) -> Merge1/1
  If Action Subsystem1/1(JerkFuncDecMax) -> Merge/2
  If/1 -> If Action Subsystem/Action
  Compare To Constant/1 -> If/1
  subjVel/1 -> If Action Subsystem1/1(subjVel)
  If/2 -> If Action Subsystem1/Action
  ObjectMovingState/1 -> Compare To Constant/1
  If Action Subsystem/1(JerkFuncDecMax) -> Merge/1

== system TosAeb/FcwAebJa_Step_sys/ALG_AEBSystem/ALG_AEBController/AEB_FuncImpManagementApp/FuncManeuArbCalc/brkFuncManeuArbCalc/axTgtReqValueCalc1/AebfullTriggerInfoCalc/LowPassFilter ==
  x : Inport
  Add : Sum
  Add1 : Sum  Inputs=+-
  Constant : Constant  Value=FiltCoef
  Constant1 : Constant  OutDataTypeStr=single
  Constant2 : Constant  Value=FiltCoef
  Delay : Delay  DelayLength=1  InitialConditionSource=Input port
      settings: InputPortMap=u0,p4
  Product : Product
  Product1 : Product
  y : Outport
  wires:
  Constant2/1 -> Add1/2
  Delay/1 -> Product1/2
  Add1/1 -> Product1/1
  Add/1 -> y/1, Delay/1
  Constant1/1 -> Add1/1
  Product/1 -> Add/1
  Constant/1 -> Product/2
  x/1 -> Delay/2, Product/1
  Product1/1 -> Add/2

== system TosAeb/FcwAebJa_Step_sys/ALG_AEBSystem/ALG_AEBController/AEB_FuncImpManagementApp/FuncManeuArbCalc/brkFuncManeuArbCalc/axTgtReqValueCalc1/AebfullTriggerInfoCalc/Subsystem ==
  AebActive : Inport
  subjVel : Inport  Port=2  OutDataTypeStr=single
  dxFrontBumper : Inport  Port=3
  vx : Inport  Port=4
  vxAbsObj : Inport  Port=5
  ax : Inport  Port=6
  Compare To Constant : simulink/Logic and Bit Operations/Compare To Constant  relop===  const=FALSE  OutDataTypeStr=boolean
  If : If  IfExpression=u1
  If Action Subsystem : Subsystem  TreatAsAtomicUnit=on
  Merge : Merge
  ObjectPredict : Subsystem
  AEB_FIM_ObjectPredicted : Outport
  wires:
  vx/1 -> ObjectPredict/3(vx)
  ax/1 -> ObjectPredict/5(ax)
  subjVel/1 -> ObjectPredict/1(subjVel)
  If/1 -> If Action Subsystem/Action
  If/2 -> ObjectPredict/Action
  Compare To Constant/1 -> If/1
  dxFrontBumper/1 -> ObjectPredict/2(dxFrontBumper)
  ObjectPredict/1(ObjectPredicted) -> Merge/2
  vxAbsObj/1 -> ObjectPredict/4(vxAbsObj)
  If Action Subsystem/1(ObjectPredicted) -> Merge/1
  Merge/1 -> AEB_FIM_ObjectPredicted/1  "AEB_FIM_ObjectPredicted"
  AebActive/1 -> Compare To Constant/1

== system TosAeb/FcwAebJa_Step_sys/ALG_AEBSystem/ALG_AEBController/AEB_FuncImpManagementApp/FuncManeuArbCalc/brkFuncManeuArbCalc/axTgtReqValueCalc1/AebfullTriggerInfoCalc/axAbsObjFiltCalc ==
  ObjectMovingState : Inport
  axAbsObj : Inport  Port=2
  Compare To Constant : simulink/Logic and Bit Operations/Compare To Constant  relop===  const=AEB_ObjectMovingState.Still  OutDataTypeStr=boolean
  Constant : Constant  Value=CNNull
  LowPassFilter1 : Subsystem  Commented=through  mask{FiltCoef=P_AEB_FIM_FiltCeof4axAbsObj}
  Switch : Switch  Criteria=u2 ~= 0
  axAbsObjFilt : Outport
  wires:
  ObjectMovingState/1 -> Compare To Constant/1
  Compare To Constant/1 -> Switch/2
  Constant/1 -> Switch/1
  axAbsObj/1 -> LowPassFilter1/1(x)
  Switch/1 -> axAbsObjFilt/1
  LowPassFilter1/1(y) -> Switch/3  "axAbsObjFilt"

== system TosAeb/FcwAebJa_Step_sys/ALG_AEBSystem/ALG_AEBController/AEB_FuncImpManagementApp/FuncManeuArbCalc/brkFuncManeuArbCalc/axTgtReqValueCalc1/Fctb_IsNeedBrakeCalc/IsNeedBrakeFlow/Distance_Cal1 ==
  subjVel : Inport
  DistanceK1 : Inport  Port=2
  f : TriggerPort  TriggerType=function-call  VariantControl=(inherit)
      settings: CopyFcn=Stateflow.SLINSF.SimulinkMan.removeBlockPreDeleteFcn  PreDeleteFcn=Stateflow.SLINSF.SimulinkMan.unsafePredeleteFcn
  Add : Sum
  Constant : Constant  Value=Ts
  Constant1 : Constant  Value=10  OutDataTypeStr=single
  Constant2 : Constant  Value=CNNull
  Max : MinMax  Function=max  Inputs=2
  Min : MinMax  Inputs=2
  Product : Product
  Distance : Outport
  wires:
  Constant/1 -> Product/2
  DistanceK1/1 -> Add/2
  Add/1 -> Min/1
  Product/1 -> Add/1
  subjVel/1 -> Product/1
  Min/1 -> Max/1
  Max/1 -> Distance/1
  Constant1/1 -> Min/2
  Constant2/1 -> Max/2

== system TosAeb/FcwAebJa_Step_sys/ALG_AEBSystem/ALG_AEBController/AEB_FuncImpManagementApp/FuncManeuArbCalc/brkFuncManeuArbCalc/axTgtReqValueCalc1/Fctb_IsNeedBrakeCalc/IsNeedBrakeFlow/Distance_Cal2 ==
  subjVel : Inport
  Fctb_AxReq : Inport  Port=2
  f : TriggerPort  TriggerType=function-call  VariantControl=(inherit)
      settings: CopyFcn=Stateflow.SLINSF.SimulinkMan.removeBlockPreDeleteFcn  PreDeleteFcn=Stateflow.SLINSF.SimulinkMan.unsafePredeleteFcn
  Compare To Constant : simulink/Logic and Bit Operations/Compare To Constant  relop=>=  const=-0.01  OutDataTypeStr=boolean
  If : If  IfExpression=u1
  If Action Subsystem : Subsystem  TreatAsAtomicUnit=on
  Merge : Merge
  Subsystem1 : Subsystem
  Distance : Outport
  wires:
  subjVel/1 -> Subsystem1/2(subjVel)
  If Action Subsystem/1(Distance2) -> Merge/1
  If/1 -> If Action Subsystem/Action
  If/2 -> Subsystem1/Action
  Subsystem1/1(Distance2) -> Merge/2
  Compare To Constant/1 -> If/1
  Fctb_AxReq/1 -> Compare To Constant/1, Subsystem1/1(Fctb_AxReq)
  Merge/1 -> Distance/1

== system TosAeb/FcwAebJa_Step_sys/ALG_AEBSystem/ALG_AEBController/AEB_FuncImpManagementApp/FuncManeuArbCalc/brkFuncManeuArbCalc/axTgtReqValueCalc1/axTgtReqValueCalc4AebControl/JerkFuncDecCalc ==
  aDesired : Inport
  FuncActvationTime : Inport  Port=2
  AebActiveFirstCycle : Inport  Port=3
  FuncActvationTimeAtStart : Inport  Port=4
  vxAtStart : Inport  Port=5
  accelMin : Inport  Port=6
  JerkFuncDecMax : Inport  Port=7
  axTgtReqValueK1 : Inport  Port=8
  Bus Creator : BusCreator  Inputs=8
  Bus Selector : BusSelector  OutputSignals=aDesired,axTgtReqValueK1,FuncActvationTime,FuncActvationTimeAtStart,vxAtStart
  Bus Selector1 : BusSelector  OutputSignals=JerkFuncDecMax,aDesired,accelMin,axTgtReqValueK1,AebActiveFirstCycle
  GradientFuncDecK1Opt : Subsystem
  JerkFuncDecCalc : Subsystem
  JerkFuncDec : Outport
  wires:
  Bus Selector1/4 -> JerkFuncDecCalc/4(axTgtReqValueK1)  "<axTgtReqValueK1>"
  Bus Selector1/3 -> JerkFuncDecCalc/3(accelMin)  "<accelMin>"
  Bus Selector1/2 -> JerkFuncDecCalc/2(aDesired)  "<aDesired>"
  Bus Selector1/1 -> JerkFuncDecCalc/1(JerkFuncDecMax)  "<JerkFuncDecMax>"
  JerkFuncDecMax/1 -> Bus Creator/7  "JerkFuncDecMax"
  Bus Selector/5 -> GradientFuncDecK1Opt/5(vxAtStart)  "<vxAtStart>"
  Bus Selector/4 -> GradientFuncDecK1Opt/4(FuncActvationTimeAtStart)  "<FuncActvationTimeAtStart>"
  Bus Selector/3 -> GradientFuncDecK1Opt/3(FuncActvationTime)  "<FuncActvationTime>"
  Bus Selector/2 -> GradientFuncDecK1Opt/2(axTgtReqValueK1)  "<axTgtReqValueK1>"
  Bus Selector/1 -> GradientFuncDecK1Opt/1(aDesired)  "<aDesired>"
  Bus Creator/1 -> Bus Selector1/1, Bus Selector/1
  axTgtReqValueK1/1 -> Bus Creator/8  "axTgtReqValueK1"
  accelMin/1 -> Bus Creator/6  "accelMin"
  vxAtStart/1 -> Bus Creator/5  "vxAtStart"
  FuncActvationTimeAtStart/1 -> Bus Creator/4  "FuncActvationTimeAtStart"
  FuncActvationTime/1 -> Bus Creator/2  "FuncActvationTime"
  aDesired/1 -> Bus Creator/1  "aDesired"
  GradientFuncDecK1Opt/2(JerkDecHoldTime) -> JerkFuncDecCalc/7(JerkDecHoldTime)
  JerkFuncDecCalc/1(JerkFuncDec) -> JerkFuncDec/1
  GradientFuncDecK1Opt/1(JerkFuncDecReset) -> JerkFuncDecCalc/6(JerkFuncDecReset)
  AebActiveFirstCycle/1 -> Bus Creator/3  "AebActiveFirstCycle"
  Bus Selector1/5 -> JerkFuncDecCalc/5(AebActiveFirstCycle)  "<AebActiveFirstCycle>"

== system TosAeb/FcwAebJa_Step_sys/ALG_AEBSystem/ALG_AEBController/AEB_FuncImpManagementApp/FuncManeuArbCalc/brkFuncManeuArbCalc/axTgtReqValueCalc1/axTgtReqValueCalc4AebControl/JerkFuncIncCalc ==
  note: Phase -> Stage
      | Achieved -> Reached/Arrived
      | Gradient -> Jerk
  axTgtReqValueK1 : Inport
  axAbsObj : Inport  Port=2
  dxFrontBumperPredicted : Inport  Port=3
  vxPredictedAtStart : Inport  Port=4
  AebActiveFirstCycle : Inport  Port=5
  aDesired : Inport  Port=6
  IncPhaseCalc : Subsystem
  JerkFuncIncCalc : Subsystem
  aErrorDesiredTgtValueCalc : Subsystem
  longBrakingFlg : Subsystem
  ReachedIncStage : Outport
  currentIncStage : Outport  Port=2
  JerkFuncInc : Outport  Port=3
  wires:
  JerkFuncIncCalc/1(JerkFuncInc) -> JerkFuncInc/1
  IncPhaseCalc/2(currentIncStage) -> currentIncStage/1
  IncPhaseCalc/1(ReachedIncStage) -> ReachedIncStage/1
  longBrakingFlg/1(longBrakingFlg) -> JerkFuncIncCalc/2(longBrakingFlg)  "AEB_FIM_longBrakingFlg"
  vxPredictedAtStart/1 -> longBrakingFlg/2(vxPredictedAtStart)
  axAbsObj/1 -> longBrakingFlg/1(axAbsObj)
  dxFrontBumperPredicted/1 -> IncPhaseCalc/2(dxFrontBumperPredicted)
  aErrorDesiredTgtValueCalc/1(aErrorDesiredTgtValue) -> IncPhaseCalc/1(aErrorDesiredTgtValue)
  aDesired/1 -> aErrorDesiredTgtValueCalc/2(aDesired)
  axTgtReqValueK1/1 -> aErrorDesiredTgtValueCalc/1(axTgtReqValueK1)
  IncPhaseCalc/3(time4IncControlling) -> JerkFuncIncCalc/1(time4IncControlling)  "AEB_FIM_time4IncControlling"
  AebActiveFirstCycle/1 -> IncPhaseCalc/3(AebActiveFirstCycle)
